simulink model "BaseEngineController_A02"  (Simulink 7.7)
settings: Solver=FixedStepDiscrete  StartTime=0.0  StopTime=10.0  RelTol=1e-3
PreLoadFcn:
  | try, eval([bdroot '_setup']); catch, eval('model_setup', ''); end
InitFcn:
  | try, eval([bdroot '_setup']); catch, eval('model_setup', ''); end
undefined symbols (not defined in the file or in its folder): ECU555, High, motohawk_build_version, motohawk_get_faults, motohawk_trunc_name, motohawk_vardec_config_group, motohawk_vardec_path, True
libraries: MotoHawk_lib x1145, AutoDoc_lib x786, motohawk_control_primitives_lib x114, motohawk_engine_lib x19, motohawk_annotations_lib x12, simulink x11, simulink_extras x1
(part 2 of 23)

== system BaseEngineController_A02/Foreground/Control/Diagnostics ==
  Control : Inport
  Sensors : Inport  Port=2
  Trigger : TriggerPort  TriggerType=function-call
  Bus Creator1 : BusCreator  Inputs=5
  Bus Selector : BusSelector  OutputSignals=Raw.RPMInst
  Constant : Constant  Value=uint8(1)
  Documentation_Calibrations_Probes_Block : AutoDoc_lib/Documentation_Calibrations_Probes_Block
  Documentation_Left_Border : AutoDoc_lib/Documentation_Left_Border  layout=Portrait
  Documentation_Right_Border : AutoDoc_lib/Documentation_Right_Border  layout=Portrait
  Documentation_Title_Block : AutoDoc_lib/Documentation_Title_Block
  Engine Overheat Fault Logic : Subsystem
  Fault Codes and States : Subsystem  TreatAsAtomicUnit=on
  Freeze Frame Trigger : Subsystem
  Freeze Frames : Subsystem
  Fuel Economy : Subsystem
  Low Oil Pressure Fault Logic : Subsystem
  Low System Voltage Fault Logic : Subsystem
  MAP Fault Logic : Subsystem
  Mux : Mux  Inputs=2
  Overspeed Protection : Subsystem  AncestorBlock=motohawk_engine_lib/Overspeed Protection
      mask Description:
      | Block allows for three levels of overspeed rev limits, and will set three fault accordingly.
      | 
      | Soft Overspeed, Medium Overspeed, and Hard Overspeed.
      mask Display:
      | patch([0 0 1 1], 0.5*[0 0.075 0.075 0], [230/255 0/255 0/255])
      | patch([0 0 1 1], 0.5*[0.025 0.05 0.05 0.025], [150/255 0/255 0/255])
      | patch([0 0 1 1], 0.5*[0 0.025 0.025 0], [0/255 0/255 0/255])
      | 
      | disp('RPM LIMITER');
  Service Intervals : Subsystem
  Terminator2 : Terminator
  Wrong Fuel Pressure Fault Logic : Subsystem
  Diagnostics : Outport
  wires:
  Service Intervals/1(HourMeter) -> Bus Creator1/2  "EngineHours"
  Bus Creator1/1 -> Diagnostics/1  "Diagnostics"
  Sensors/1 -> Bus Selector/1, Engine Overheat Fault Logic/1(Sensors), MAP Fault Logic/1(Sensors), Low Oil Pressure Fault Logic/1(Sensors), Low System Voltage Fault Logic/1(Sensors), Wrong Fuel Pressure Fault Logic/1(Sensors), Mux/2, Fuel Economy/1(Sensors)
  Control/1 -> Terminator2/1, Mux/1, Fuel Economy/2(Controller)  "Control"
  Bus Selector/1 -> Overspeed Protection/1(RPM)  "<RPMInst>"
  Freeze Frame Trigger/1(IDs) -> Freeze Frames/2(IDs)
  Freeze Frame Trigger/2(Trigger) -> Freeze Frames/3(Trigger)
  Constant/1 -> Fault Codes and States/1(IC)
  Mux/1 -> Freeze Frames/1(Data)
  Low Oil Pressure Fault Logic/1(LowOilPres) -> Bus Creator1/1  "LowOilPres"
  Engine Overheat Fault Logic/1(Overheat_Warning) -> Bus Creator1/3  "Overheat_Warning"
  Fuel Economy/1(FuelEconomy_instantaneous) -> Bus Creator1/4  "FuelEconomy_instantaneous"
  Fuel Economy/2(FuelEconomy_filtered) -> Bus Creator1/5  "FuelEconomy_filtered"

== system BaseEngineController_A02/Foreground/Control/VirtualSensors ==
  Raw : Inport
  AirCalcs : Subsystem
  Bus Creator : BusCreator  Inputs=6
  Documentation_Calibrations_Probes_Block : AutoDoc_lib/Documentation_Calibrations_Probes_Block
  Documentation_Left_Border : AutoDoc_lib/Documentation_Left_Border  layout=Portrait
  Documentation_Right_Border : AutoDoc_lib/Documentation_Right_Border  layout=Portrait
  Documentation_Title_Block : AutoDoc_lib/Documentation_Title_Block
  LoadBasedCalcs : Subsystem
  Raw Indexes : Subsystem
  RawProcessed : Subsystem
  Virtual Indexes : Subsystem
  Sensors : Outport
  wires:
  Raw/1 -> RawProcessed/1(Raw), Raw Indexes/1(Raw), AirCalcs/2(Raw), LoadBasedCalcs/2(Raw), Bus Creator/1  "Raw"
  Bus Creator/1 -> Sensors/1  "SynthSensors"
  RawProcessed/1(RawProcessed) -> Bus Creator/2, Virtual Indexes/3(RawProcessed)  "RawProcessed"
  Raw Indexes/1(RawIndexes) -> Bus Creator/3, AirCalcs/1(RawIndexes), RawProcessed/2(Indexes)  "RawIndexes"
  AirCalcs/1(AirCalcs) -> Bus Creator/4, LoadBasedCalcs/1(AirCals), Virtual Indexes/2(AirCalcs)  "AirCalcs"
  LoadBasedCalcs/1(TorqueCalcs) -> Bus Creator/5, Virtual Indexes/1(TorqueCalcs)  "TorqueCalcs"
  Virtual Indexes/1(VirtualIndexes) -> Bus Creator/6  "VirtualIndexes"

== system BaseEngineController_A02/Foreground/Inputs/CAN Inputs ==
  112 : Subsystem
  Bus Creator : BusCreator  Inputs=2
  Phoenix : Subsystem
  Out1 : Outport
  wires:
  112/1(Out1) -> Bus Creator/1  "112"
  Phoenix/1(Out1) -> Bus Creator/2  "Phoenix"
  Bus Creator/1 -> Out1/1

== system BaseEngineController_A02/Foreground/Inputs/Digital ==
  Bus Creator : BusCreator  Inputs=3
  Constant : Constant  Value=true
  Data Type Conversion : DataTypeConversion
  Documentation_Calibrations_Probes_Block : AutoDoc_lib/Documentation_Calibrations_Probes_Block
  Documentation_Left_Border : AutoDoc_lib/Documentation_Left_Border  layout=Portrait
  Documentation_Right_Border : AutoDoc_lib/Documentation_Right_Border  layout=Portrait
  Documentation_Title_Block : AutoDoc_lib/Documentation_Title_Block
  Gain : Gain  Gain=.01
  MAF_Sensor : motohawk_engine_lib/Non-Linear Sensor Characterization (tbl)  SystemSampleTime=-1  nam='MAF'  prefix='MAF'  units='g/s'  inputunits='Hz'  parent_group=motohawk_vardec_path(gcb)  tblsize=40  samp_time=-1
  Terminator1 : Terminator
  Terminator4 : Terminator
  motohawk_callout1 : motohawk_annotations_lib/motohawk_callout1  BlockType_='Mototron_harea'  desc='\fontsize{12}Read from H/W'  edit_text=Compute the mean forward body acceleration.Worry about rounding.  edit_as=255
  motohawk_callout2 : motohawk_annotations_lib/motohawk_callout1  BlockType_='Mototron_harea'  desc='\fontsize{12}Convert to Engineering Units'  edit_text=Compute the mean forward body acceleration.Worry about rounding.  edit_as=255
  motohawk_callout3 : motohawk_annotations_lib/motohawk_callout1  BlockType_='Mototron_harea'  desc='\fontsize{12}Create a Bus of Sensors'  edit_text=Compute the mean forward body acceleration.Worry about rounding.  edit_as=255
  motohawk_data_read : MotoHawk_lib/Data Storage Blocks/motohawk_data_read  source=Lookup By Name  structure=Scalar  operation=Read scalar from location by index  sz_vec=4  sz_row=2  sz_col=3  typ=boolean  nam='ESTOP'  checkNull=on  struct_inst_name='MyData'  struct_nam='MyStruct'
  motohawk_frequency_in2 : MotoHawk_lib/Advanced Digital I//O/motohawk_frequency_in  res_name=[]  text_resource=(None)  code_resource=RES_DG1M  val_resource=-1  target=ECU555-80  resource_enum_str=(None)=-1,DG1M=93,DG2M=94,DG4M=96,CNK=100  ipwm=Perform Duty Cycle Measument  itrig=off  Nmax=8  edge=Falling Edge  alias=Disabled  min_freq=10  sig=Digital Input  use_vardec=on  nam='MAF_Pin'  read_level=1  write_level=1  group='System | I/O Pins | Inputs Config'  cal_group='System | I/O Pins | Frequency In Config'
  motohawk_override_abs1 : MotoHawk_lib/Calibration & Probing Blocks/motohawk_override_abs  SystemSampleTime=-1  parent_name='ESTOP_Ovr'  parent_val=0  show_more=off  name_source=Use Parameter  parent_read_level=1  parent_override_level=1  view_as=Number  enum_struct={'Disabled', 'Enabled'}  use_help_units=off  parent_help=''  parent_units=''  use_min_max=off  parent_min=-inf  parent_max=inf  use_precision=off  parent_precision=''  parent_gain=1  parent_offset=0  parent_exp=1  use_mototune_group=off  parent_group=motohawk_vardec_path(gcb)
  motohawk_probe1 : MotoHawk_lib/Calibration & Probing Blocks/motohawk_probe  nam='ESTOP_Prb'  show_more=off  name_source=Use Parameter  read_level=1  view_as=Number  enum_struct={'Disabled', 'Enabled'}  vector_style=Wide Row  use_help_units=off  help=''  units=''  use_header_enum=off  row_header_enum={}  col_header_enum={}  use_precision=off  precision=''  gain=1  offset=0  exp=1  use_mototune_group=off  mototune_group=motohawk_vardec_path(gcb)
  SensorBus : Outport
  wires:
  Bus Creator/1 -> SensorBus/1  "Digital"
  Constant/1 -> Bus Creator/1  "GearState"
  motohawk_frequency_in2/1 -> Data Type Conversion/1
  MAF_Sensor/1 -> Bus Creator/2  "MAF"
  MAF_Sensor/2 -> Terminator4/1
  Data Type Conversion/1 -> Gain/1
  Gain/1 -> MAF_Sensor/1
  motohawk_frequency_in2/2 -> Terminator1/1
  motohawk_data_read/1 -> motohawk_override_abs1/1, motohawk_probe1/1  "EStop"
  motohawk_override_abs1/1 -> Bus Creator/3  "ESTOP"

== system BaseEngineController_A02/Foreground/Inputs/EngineSpeed ==
  note: Set PseudoRPM from MotoTune
      | to a non-zero value to cause
      | simulated RPM
  Constant : Constant  Value=0  OutDataTypeStr=Inherit: Inherit via back propagation
  Data Type Conversion : DataTypeConversion  SaturateOnIntegerOverflow=on  RndMeth=Zero
  Data Type Conversion1 : DataTypeConversion  SaturateOnIntegerOverflow=on  RndMeth=Zero
  Data Type Conversion3 : DataTypeConversion  RndMeth=Zero
  Documentation_Calibrations_Probes_Block : AutoDoc_lib/Documentation_Calibrations_Probes_Block
  Documentation_Left_Border : AutoDoc_lib/Documentation_Left_Border  layout=Portrait
  Documentation_Right_Border : AutoDoc_lib/Documentation_Right_Border  layout=Portrait
  Documentation_Title_Block : AutoDoc_lib/Documentation_Title_Block
  Encoder Diagnostics : Subsystem
  First Order Low Pass (Tunable)1 : motohawk_control_primitives_lib/First Order Low Pass (Tunable)  SystemSampleTime=-1  nam='RPMFiltConst'  samp_time=-1  group=motohawk_vardec_path(gcb)
  Gain : Gain  Gain=16  SaturateOnIntegerOverflow=on  OutDataTypeStr=Inherit: Same as input  ParamDataTypeStr=Inherit: Same as input
  Relational Operator : RelationalOperator  Operator=~=  ZeroCross=off  InputSameDT=on
  motohawk_calibration : MotoHawk_lib/Calibration & Probing Blocks/motohawk_calibration  nam='PseudoRPM'  val=0  storage=Display  show_more=on  name_source=Use Parameter  data_type=Inherit via back propagation  read_level=1  write_level=1  ovr_cals=on  view_as=Number  enum_struct={'Disabled', 'Enabled'}  vector_style=Wide Row  use_help_units=off  help=''  units=''  use_header_enum=off  row_header_enum={}  col_header_enum={}  use_min_max=off  min_val=-inf  max_val=inf  use_precision=off  precision=''  gain=1  offset=0  exp=1  use_mototune_group=on  mototune_group=motohawk_vardec_path(gcb)
  motohawk_calibration1 : MotoHawk_lib/Calibration & Probing Blocks/motohawk_calibration  nam='EngineToEncoderOffset'  val=0  storage=Calibration  show_more=on  name_source=Use Parameter  data_type=Inherit from 'Default Value'  read_level=1  write_level=1  ovr_cals=on  view_as=Number  enum_struct={'Disabled', 'Enabled'}  vector_style=Wide Row  use_help_units=on  help=''  units=''  use_header_enum=off  row_header_enum={}  col_header_enum={}  use_min_max=off  min_val=-inf  max_val=inf  use_precision=off  precision=''  gain=1  offset=0  exp=1  use_mototune_group=on  mototune_group=motohawk_vardec_path(gcb)
  motohawk_encoder_average_rpm : MotoHawk_lib/Encoder Blocks/motohawk_encoder_average_rpm
  motohawk_encoder_def : MotoHawk_lib/Encoder Blocks/motohawk_encoder_def  encoder_type=24 Minus 1 - 4-Stroke - No CAM  interface=VR Crank  sync=Rising Edge  crnkpullup=Strong Pullup  camsync=Rising Edge  campullup=Strong Pullup  angle_offset=27  sec_tdcoffset=-34.3  cam_delay=0  teeth_after_cam=-1  ignore_teeth=6  ignore_delay=100  angles=[0 270 360 630]  cnk_align_with_cam=off  adaptive_vr_cnk=off  adaptive_vr_cam=on  cal_cylinders=on  max_cylinders=8  num_cylinders=2  read_level=1  write_level=1  ovr_cals=on  group=motohawk_vardec_path(gcb)
  motohawk_encoder_fault : MotoHawk_lib/Encoder Blocks/motohawk_encoder_fault
  motohawk_encoder_instant_rpm : MotoHawk_lib/Encoder Blocks/motohawk_encoder_instant_rpm
  motohawk_encoder_offset : MotoHawk_lib/Encoder Blocks/motohawk_encoder_offset
  motohawk_encoder_pseudo : MotoHawk_lib/Encoder Blocks/motohawk_encoder_pseudo
  motohawk_encoder_state : MotoHawk_lib/Encoder Blocks/motohawk_encoder_state
  motohawk_probe : MotoHawk_lib/Calibration & Probing Blocks/motohawk_probe  nam='RPMInst'  show_more=on  name_source=Use Parameter  read_level=1  view_as=Number  enum_struct={'Disabled', 'Enabled'}  vector_style=Wide Row  use_help_units=off  help=''  units=''  use_header_enum=off  row_header_enum={}  col_header_enum={}  use_precision=off  precision=''  gain=1  offset=0  exp=1  use_mototune_group=on  mototune_group=motohawk_vardec_path(gcb)
  motohawk_probe1 : MotoHawk_lib/Calibration & Probing Blocks/motohawk_probe  nam='RPM'  show_more=on  name_source=Use Parameter  read_level=1  view_as=Number  enum_struct={'Disabled', 'Enabled'}  vector_style=Wide Row  use_help_units=on  help=''  units=''  use_header_enum=off  row_header_enum={}  col_header_enum={}  use_precision=on  precision=''  gain=1  offset=0  exp=1  use_mototune_group=on  mototune_group=motohawk_vardec_path(gcb)
  motohawk_probe2 : MotoHawk_lib/Calibration & Probing Blocks/motohawk_probe  nam='EncoderState'  show_more=on  name_source=Use Parameter  read_level=1  view_as=Enumeration  enum_struct={'zero','ZERO_SPEED', 'POSN_UNKNOWN','ENC_SYNC_CAM_UNKNOWN','ENC_SYNC_CAM_SYNC'}  vector_style=Wide Row  use_help_units=off  help=''  units=''  use_header_enum=off  row_header_enum={}  col_header_enum={}  use_precision=off  precision=''  gain=1  offset=0  exp=1  use_mototune_group=on  mototune_group=motohawk_vardec_path(gcb)
  RPMInst : Outport
  RPM : Outport  Port=2
  wires:
  First Order Low Pass (Tunable)1/1 -> motohawk_probe1/1, RPM/1  "RPM"
  motohawk_encoder_fault/1 -> Encoder Diagnostics/1(Encoder Fault)
  motohawk_encoder_state/1 -> motohawk_probe2/1
  Data Type Conversion3/1 -> motohawk_encoder_offset/1
  Gain/1 -> Data Type Conversion3/1
  motohawk_calibration1/1 -> Gain/1
  Data Type Conversion1/1 -> motohawk_probe/1, RPMInst/1  "RPMInst"
  Data Type Conversion/1 -> First Order Low Pass (Tunable)1/1  "RPM"
  Constant/1 -> Relational Operator/1
  Relational Operator/1 -> motohawk_encoder_pseudo/1
  motohawk_calibration/1 -> Relational Operator/2, motohawk_encoder_pseudo/2
  motohawk_encoder_average_rpm/1 -> Data Type Conversion/1  "RPM"
  motohawk_encoder_instant_rpm/1 -> Data Type Conversion1/1  "RPMInst"

== system BaseEngineController_A02/Foreground/Inputs/LinearSensors ==
  Bus Creator3 : BusCreator  Inputs=9
  Documentation_Calibrations_Probes_Block : AutoDoc_lib/Documentation_Calibrations_Probes_Block
  Documentation_Left_Border : AutoDoc_lib/Documentation_Left_Border  layout=Portrait
  Documentation_Right_Border : AutoDoc_lib/Documentation_Right_Border  layout=Portrait
  Documentation_Title_Block : AutoDoc_lib/Documentation_Title_Block
  EGRSensor1 : motohawk_engine_lib/Linear Sensor Characterization  SystemSampleTime=-1  nam='EGRSense'  prefix='EGRSense'  units='%'  inputunits='V'  parent_group=motohawk_vardec_path(gcb)  samp_time=-1
  FuelOxygenationSensor : motohawk_engine_lib/Linear Sensor Characterization  SystemSampleTime=-1  nam='FuelSens'  prefix='FuelSens'  units='%'  inputunits='Hz'  parent_group=motohawk_vardec_path(gcb)  samp_time=-1
  FuelPres : motohawk_engine_lib/Linear Sensor Characterization  SystemSampleTime=-1  nam='FuelPres'  prefix='FuelPres'  units='kPa Gauge'  inputunits='ADC'  parent_group=motohawk_vardec_path(gcb)  samp_time=-1
  Gain : Gain  Gain=100  OutDataTypeStr=double
  MAP : motohawk_engine_lib/Linear Sensor Characterization  SystemSampleTime=-1  nam='MAP'  prefix='MAP'  units='kPa'  inputunits='ADC'  parent_group=motohawk_vardec_path(gcb)  samp_time=-1
  MAPTime : motohawk_engine_lib/Linear Sensor Characterization  SystemSampleTime=-1  nam='MAPTime'  prefix='MAPTime'  units='kPa'  inputunits='ADC'  parent_group=motohawk_vardec_path(gcb)  samp_time=-1
  MAP_Crank_Synchronous : Subsystem
      mask Display: disp('Crank-Synchronous MAP');
  OilPres : motohawk_engine_lib/Linear Sensor Characterization  SystemSampleTime=-1  nam='OilPres'  prefix='OilPres'  units='psi'  inputunits='ADC'  parent_group=motohawk_vardec_path(gcb)  samp_time=-1
  SensVolts : motohawk_engine_lib/Linear Sensor Characterization  SystemSampleTime=-1  nam='SensVolt'  prefix='SensVolt'  units='Volts'  inputunits='ADC'  parent_group=motohawk_vardec_path(gcb)  samp_time=-1
  SysVolts : motohawk_engine_lib/Linear Sensor Characterization  SystemSampleTime=-1  nam='SysVolt'  prefix='SysVolt'  units='Volts'  inputunits='ADC'  parent_group=motohawk_vardec_path(gcb)  samp_time=-1
  Terminator{1..7} (7 alike) : Terminator
  motohawk_ain1 : MotoHawk_lib/Analog I//O Blocks/motohawk_ain  res_name=[]  text_resource=(None)  code_resource=RES_ECUP  val_resource=-1  target=ECU555-80  resource_enum_str=(None)=-1,ECUP=53,DRVP=54,XDRP=55,AN1M=56,AN2M=57,AN3M=58,AN4M=59,AN5M=60,AN6M=61,AN7M=62,AN8M=63,AN9M=64,AN10M=65,AN11M=66,AN12M=67,AN13M=68,AN14M=69,AN15M=70,AN16M=71,AN17M=72,AN18M=73,AN19M=74,O2A=90,O2B=91,XDRP_B=92  altref=5V Reference  typ=uint16  num_adc_bits=10  use_stat=off  use_vardec=on  nam='OilPresPin'  read_level=1  write_level=1  group='System | I/O Pins | Inputs Config'
  motohawk_ain14 : MotoHawk_lib/Analog I//O Blocks/motohawk_ain  res_name=[]  text_resource=DRVP  code_resource=RES_ECUP  val_resource=-1  target=ECU555-80  resource_enum_str=(None)=-1,ECUP=53,DRVP=54,XDRP=55,AN1M=56,AN2M=57,AN3M=58,AN4M=59,AN5M=60,AN6M=61,AN7M=62,AN8M=63,AN9M=64,AN10M=65,AN11M=66,AN12M=67,AN13M=68,AN14M=69,AN15M=70,AN16M=71,AN17M=72,AN18M=73,AN19M=74,O2A=90,O2B=91,XDRP_B=92  altref=5V Reference  typ=uint16  num_adc_bits=10  use_stat=off  use_vardec=on  nam='SysVoltage'  read_level=1  write_level=1  group='System | I/O Pins | Inputs Config'
  motohawk_ain2 : MotoHawk_lib/Analog I//O Blocks/motohawk_ain  res_name=[]  text_resource=(None)  code_resource=RES_ECUP  val_resource=-1  target=ECU555-80  resource_enum_str=(None)=-1,ECUP=53,DRVP=54,XDRP=55,AN1M=56,AN2M=57,AN3M=58,AN4M=59,AN5M=60,AN6M=61,AN7M=62,AN8M=63,AN9M=64,AN10M=65,AN11M=66,AN12M=67,AN13M=68,AN14M=69,AN15M=70,AN16M=71,AN17M=72,AN18M=73,AN19M=74,O2A=90,O2B=91,XDRP_B=92  altref=5V Reference  typ=uint16  num_adc_bits=10  use_stat=off  use_vardec=on  nam='FuelPresPin'  read_level=1  write_level=1  group='System | I/O Pins | Inputs Config'
  motohawk_ain3 : MotoHawk_lib/Analog I//O Blocks/motohawk_ain  res_name=[]  text_resource=XDRP  code_resource=RES_ECUP  val_resource=-1  target=ECU555-80  resource_enum_str=(None)=-1,ECUP=53,DRVP=54,XDRP=55,AN1M=56,AN2M=57,AN3M=58,AN4M=59,AN5M=60,AN6M=61,AN7M=62,AN8M=63,AN9M=64,AN10M=65,AN11M=66,AN12M=67,AN13M=68,AN14M=69,AN15M=70,AN16M=71,AN17M=72,AN18M=73,AN19M=74,O2A=90,O2B=91,XDRP_B=92  altref=5V Reference  typ=uint16  num_adc_bits=10  use_stat=off  use_vardec=on  nam='SensVoltSensorPin'  read_level=1  write_level=1  group='System | I/O Pins | Inputs Config'
  motohawk_ain4 : MotoHawk_lib/Analog I//O Blocks/motohawk_ain  res_name=[]  text_resource=(None)  code_resource=RES_ECUP  val_resource=-1  target=ECU555-80  resource_enum_str=(None)=-1,ECUP=53,DRVP=54,XDRP=55,AN1M=56,AN2M=57,AN3M=58,AN4M=59,AN5M=60,AN6M=61,AN7M=62,AN8M=63,AN9M=64,AN10M=65,AN11M=66,AN12M=67,AN13M=68,AN14M=69,AN15M=70,AN16M=71,AN17M=72,AN18M=73,AN19M=74,O2A=90,O2B=91,XDRP_B=92  altref=5V Reference  typ=uint16  num_adc_bits=10  use_stat=off  use_vardec=on  nam='EGRPin'  read_level=1  write_level=1  group='System | I/O Pins | Inputs Config'
  motohawk_ain_read1 : MotoHawk_lib/Analog I//O Blocks/motohawk_ain_read  nam='MAPSensorPin'  typ=uint16
  motohawk_callout1 : motohawk_annotations_lib/motohawk_callout1  BlockType_='Mototron_harea'  desc='\fontsize{12}Read from H/W'  edit_text=Compute the mean forward body acceleration.Worry about rounding.  edit_as=255
  motohawk_callout2 : motohawk_annotations_lib/motohawk_callout1  BlockType_='Mototron_harea'  desc='\fontsize{12}Convert to Engineering Units'  edit_text=Compute the mean forward body acceleration.Worry about rounding.  edit_as=255
  motohawk_callout3 : motohawk_annotations_lib/motohawk_callout1  BlockType_='Mototron_harea'  desc='\fontsize{12}Create a Bus of Sensors'  edit_text=Compute the mean forward body acceleration.Worry about rounding.  edit_as=255
  motohawk_frequency_in : MotoHawk_lib/Advanced Digital I//O/motohawk_frequency_in  res_name=[]  text_resource=DG1M  code_resource=RES_DG1M  val_resource=-1  target=ECU555-80  resource_enum_str=(None)=-1,DG1M=93,DG2M=94,DG4M=96,CNK=100  ipwm=No Duty Cycle Measurement  itrig=off  Nmax=10  edge=Rising Edge  alias=Disabled  min_freq=10  sig=Digital Input  use_vardec=on  nam='FuelSensorPin'  read_level=1  write_level=1  group='System | I/O Pins | Inputs Config'  cal_group='System | I/O Pins | Frequency In Config'
  SensorBus : Outport
  wires:
  Bus Creator3/1 -> SensorBus/1  "LinearSensors"
  motohawk_ain_read1/1 -> MAPTime/1
  MAP_Crank_Synchronous/1(ADC) -> MAP/1
  SysVolts/2 -> Terminator4/1
  SensVolts/2 -> Terminator3/1
  MAP/2 -> Terminator2/1
  MAPTime/2 -> Bus Creator3/5  "MAP_Time_Raw"
  MAPTime/1 -> Bus Creator3/4  "Map_Time"
  MAP/1 -> Bus Creator3/3  "MAP_Angular"
  SensVolts/1 -> Bus Creator3/2  "SensVolt"
  motohawk_ain3/1 -> SensVolts/1
  SysVolts/1 -> Bus Creator3/1  "SysVolt"
  motohawk_ain14/1 -> SysVolts/1
  motohawk_ain1/1 -> OilPres/1
  OilPres/2 -> Terminator1/1
  OilPres/1 -> Bus Creator3/6  "OilPres"
  motohawk_frequency_in/1 -> Gain/1
  FuelOxygenationSensor/2 -> Terminator6/1
  motohawk_ain2/1 -> FuelPres/1
  FuelPres/2 -> Terminator7/1
  FuelOxygenationSensor/1 -> Bus Creator3/7  "FuelSense"
  FuelPres/1 -> Bus Creator3/8  "FuelPres"
  Gain/1 -> FuelOxygenationSensor/1
  EGRSensor1/2 -> Terminator5/1
  motohawk_ain4/1 -> EGRSensor1/1
  EGRSensor1/1 -> Bus Creator3/9  "EGRPos"

== system BaseEngineController_A02/Foreground/Inputs/NonLinearSensors ==
  Bus Creator1 : BusCreator  Inputs=6
  Documentation_Calibrations_Probes_Block : AutoDoc_lib/Documentation_Calibrations_Probes_Block
  Documentation_Left_Border : AutoDoc_lib/Documentation_Left_Border  layout=Portrait
  Documentation_Right_Border : AutoDoc_lib/Documentation_Right_Border  layout=Portrait
  Documentation_Title_Block : AutoDoc_lib/Documentation_Title_Block
  O2_PostCat : Subsystem  AncestorBlock=motohawk_engine_lib/Non-Linear Sensor Characterization (tbl)  mask(Non-Linear Sensor Characterization (tbl)){nam='O2_PostCat', prefix='O2_PostCat', units='Equiv Ratio', inputunits='ADC', parent_group=motohawk_vardec_path(gcb), bp=[12 17 25 30 37 42 48 71 100 130 168 202 256 281 300 323 338 361 379 380], data=[0.75 0.86 0.93 0.96 0.98 0.99 0.995 0.998 0.999 1 1.001 1.002 1.005 1.01 1.02 1.04 1.06 1.1 1.15 1.25], min=1, max=425, default=1, filt_const=.005, samp_time=-1}
      mask prompts: nam "Name"; units "Output Units"; inputunits "Input Units"; parent_group "MotoTune Group"; bp "Breakpoints"; data "Table Data"; min "Min Fault Thresh"; max "Max Fault Thresh"; default "Initial Default Value When Fault Suspected"; filt_const "1st-Order Filter Constant"; samp_time "Sample Time"
      mask help:
      | Converts from A/D Counts to Engineering Units using a 1-D Table Lookup.
      | Saturates the converted value around Min and Max values and triggers a Fault when out of range.
      mask init:
      | lbl = ['Non-Linear Sensor (tbl): ' nam ' [' units ']'];
      | group = [parent_group ' | ' nam];
      settings: RTWSystemCode=Function  RTWFcnNameOpts=Use subsystem name  RTWFileNameOpts=Use function name
      mask Display:
      | patch([0 0 1 1], [0 0.075 0.075 0], [230/255 0/255 0/255])
      | patch([0 0 1 1], [0.025 0.05 0.05 0.025], [150/255 0/255 0/255])
      | patch([0 0 1 1], [0 0.025 0.025 0], [0/255 0/255 0/255])
      | 
      | disp(lbl);
  Temp Sensors : Subsystem  TreatAsAtomicUnit=on
  Terminator2 : Terminator
  motohawk_ain3 : MotoHawk_lib/Analog I//O Blocks/motohawk_ain  res_name=[]  text_resource=AN12M  code_resource=RES_ECUP  val_resource=-1  target=ECU555-80  resource_enum_str=(None)=-1,ECUP=53,DRVP=54,XDRP=55,AN1M=56,AN2M=57,AN3M=58,AN4M=59,AN5M=60,AN6M=61,AN7M=62,AN8M=63,AN9M=64,AN10M=65,AN11M=66,AN12M=67,AN13M=68,AN14M=69,AN15M=70,AN16M=71,AN17M=72,AN18M=73,AN19M=74,O2A=90,O2B=91,XDRP_B=92  altref=5V Reference  typ=uint16  num_adc_bits=10  use_stat=off  use_vardec=on  nam='PostO2Pin'  read_level=1  write_level=1  group='System | I/O Pins | Inputs Config'
  motohawk_callout1 : motohawk_annotations_lib/motohawk_callout1  BlockType_='Mototron_harea'  desc='\fontsize{12}Read from H/W'  edit_text=Compute the mean forward body acceleration.Worry about rounding.  edit_as=255
  motohawk_callout2 : motohawk_annotations_lib/motohawk_callout1  BlockType_='Mototron_harea'  desc='\fontsize{12}Convert to Engineering Units'  edit_text=Compute the mean forward body acceleration.Worry about rounding.  edit_as=255
  motohawk_callout3 : motohawk_annotations_lib/motohawk_callout1  BlockType_='Mototron_harea'  desc='\fontsize{12}Create a Bus of Sensors'  edit_text=Compute the mean forward body acceleration.Worry about rounding.  edit_as=255
  motohawk_trigger : MotoHawk_lib/Trigger Blocks/motohawk_trigger  Tag=Trigger  trigger=FGND_5XRTI_PERIODIC  function_name=Trigger_FGND_5XRTI_PERIODIC_8397p0001  priority_order=1  inherited=on  run_disabled=off  override_app_monitor=off  target=ECU555_80
  SensorBus : Outport
  wires:
  Bus Creator1/1 -> SensorBus/1  "NonLinearSensors"
  Temp Sensors/2(ECT_Raw) -> Bus Creator1/2  "ECT_Raw"
  Temp Sensors/4(IAT_Raw) -> Bus Creator1/4  "IAT_Raw"
  Temp Sensors/3(IAT) -> Bus Creator1/3  "IAT"
  Temp Sensors/1(ECT) -> Bus Creator1/1  "ECT"
  motohawk_ain3/1 -> O2_PostCat/1(ADC)
  O2_PostCat/2(EngUnitsRaw) -> Terminator2/1
  motohawk_trigger/1 -> Temp Sensors/Trigger
  O2_PostCat/1(EngUnitsFilt) -> Bus Creator1/5  "O2_PostCat"
  O2_PostCat/3(O2_ADC) -> Bus Creator1/6  "O2_PostCat_ADC"

== system BaseEngineController_A02/Foreground/Inputs/PotentiometerSensors ==
  Bus Creator1 : BusCreator
  Constant4 : Constant  Value=false
  Constant5 : Constant  Value=false
  Documentation_Calibrations_Probes_Block : AutoDoc_lib/Documentation_Calibrations_Probes_Block
  Documentation_Left_Border : AutoDoc_lib/Documentation_Left_Border  layout=Portrait
  Documentation_Right_Border : AutoDoc_lib/Documentation_Right_Border  layout=Portrait
  Documentation_Title_Block : AutoDoc_lib/Documentation_Title_Block
  TPS1 : motohawk_engine_lib/Linear Sensor Characterization w Adapt  SystemSampleTime=-1  nam='TPS1'  prefix='TPS1'  samp_time=-1  parent_group=motohawk_vardec_path(gcb)
  TPS2 : motohawk_engine_lib/Linear Sensor Characterization w Adapt  SystemSampleTime=-1  nam='TPS2'  prefix='TPS2'  samp_time=-1  parent_group=motohawk_vardec_path(gcb)
  Terminator10 : Terminator
  Terminator11 : Terminator
  motohawk_ain6 : MotoHawk_lib/Analog I//O Blocks/motohawk_ain  res_name=[]  text_resource=AN2M  code_resource=RES_ECUP  val_resource=-1  target=ECU555-80  resource_enum_str=(None)=-1,ECUP=53,DRVP=54,XDRP=55,AN1M=56,AN2M=57,AN3M=58,AN4M=59,AN5M=60,AN6M=61,AN7M=62,AN8M=63,AN9M=64,AN10M=65,AN11M=66,AN12M=67,AN13M=68,AN14M=69,AN15M=70,AN16M=71,AN17M=72,AN18M=73,AN19M=74,O2A=90,O2B=91,XDRP_B=92  altref=5V Reference  typ=uint16  num_adc_bits=10  use_stat=off  use_vardec=on  nam='TPS1AnalogInput'  read_level=1  write_level=1  group=motohawk_vardec_path(gcb)
  motohawk_ain9 : MotoHawk_lib/Analog I//O Blocks/motohawk_ain  res_name=[]  text_resource=AN3M  code_resource=RES_ECUP  val_resource=-1  target=ECU555-80  resource_enum_str=(None)=-1,ECUP=53,DRVP=54,XDRP=55,AN1M=56,AN2M=57,AN3M=58,AN4M=59,AN5M=60,AN6M=61,AN7M=62,AN8M=63,AN9M=64,AN10M=65,AN11M=66,AN12M=67,AN13M=68,AN14M=69,AN15M=70,AN16M=71,AN17M=72,AN18M=73,AN19M=74,O2A=90,O2B=91,XDRP_B=92  altref=5V Reference  typ=uint16  num_adc_bits=10  use_stat=off  use_vardec=on  nam='TPS2AnalogInput'  read_level=1  write_level=1  group=motohawk_vardec_path(gcb)
  motohawk_callout1 : motohawk_annotations_lib/motohawk_callout1  BlockType_='Mototron_harea'  desc='\fontsize{12}Read from H/W'  edit_text=Compute the mean forward body acceleration.Worry about rounding.  edit_as=255
  motohawk_callout2 : motohawk_annotations_lib/motohawk_callout1  BlockType_='Mototron_harea'  desc='\fontsize{12}Convert to Engineering Units'  edit_text=Compute the mean forward body acceleration.Worry about rounding.  edit_as=255
  motohawk_callout3 : motohawk_annotations_lib/motohawk_callout1  BlockType_='Mototron_harea'  desc='\fontsize{12}Create a Bus of Sensors'  edit_text=Compute the mean forward body acceleration.Worry about rounding.  edit_as=255
  motohawk_data_read : MotoHawk_lib/Data Storage Blocks/motohawk_data_read  source=Lookup By Name  structure=Scalar  operation=Read scalar from location by index  sz_vec=4  sz_row=2  sz_col=3  typ=boolean  nam='TPSAdaptLoRequest'  checkNull=on  struct_inst_name='Struct1'  struct_nam='MyStruct'
  motohawk_data_read1 : MotoHawk_lib/Data Storage Blocks/motohawk_data_read  source=Lookup By Name  structure=Scalar  operation=Read scalar from location by index  sz_vec=4  sz_row=2  sz_col=3  typ=double  nam='APP'  checkNull=on  struct_inst_name='MyData'  struct_nam='MyStruct'
  motohawk_override_abs1 : MotoHawk_lib/Calibration & Probing Blocks/motohawk_override_abs  SystemSampleTime=-1  parent_name='APP2_Ovr'  parent_val=0  show_more=off  name_source=Use Parameter  parent_read_level=1  parent_override_level=1  view_as=Number  enum_struct={'Disabled', 'Enabled'}  use_help_units=off  parent_help=''  parent_units=''  use_min_max=off  parent_min=-inf  parent_max=inf  use_precision=off  parent_precision=''  parent_gain=1  parent_offset=0  parent_exp=1  use_mototune_group=off  parent_group=motohawk_vardec_path(gcb)
  motohawk_override_abs13 : MotoHawk_lib/Calibration & Probing Blocks/motohawk_override_abs  SystemSampleTime=-1  parent_name='APP1_Ovr'  parent_val=0  show_more=off  name_source=Use Parameter  parent_read_level=1  parent_override_level=1  view_as=Number  enum_struct={'Disabled', 'Enabled'}  use_help_units=off  parent_help=''  parent_units=''  use_min_max=off  parent_min=-inf  parent_max=inf  use_precision=off  parent_precision=''  parent_gain=1  parent_offset=0  parent_exp=1  use_mototune_group=off  parent_group=motohawk_vardec_path(gcb)
  motohawk_probe1 : MotoHawk_lib/Calibration & Probing Blocks/motohawk_probe  nam='APP2_Prb'  show_more=off  name_source=Use Parameter  read_level=1  view_as=Number  enum_struct={'Disabled', 'Enabled'}  vector_style=Wide Row  use_help_units=off  help=''  units=''  use_header_enum=off  row_header_enum={}  col_header_enum={}  use_precision=off  precision=''  gain=1  offset=0  exp=1  use_mototune_group=off  mototune_group=motohawk_vardec_path(gcb)
  motohawk_probe13 : MotoHawk_lib/Calibration & Probing Blocks/motohawk_probe  nam='APP1_Prb'  show_more=off  name_source=Use Parameter  read_level=1  view_as=Number  enum_struct={'Disabled', 'Enabled'}  vector_style=Wide Row  use_help_units=off  help=''  units=''  use_header_enum=off  row_header_enum={}  col_header_enum={}  use_precision=off  precision=''  gain=1  offset=0  exp=1  use_mototune_group=off  mototune_group=motohawk_vardec_path(gcb)
  SensorBus : Outport
  wires:
  Constant5/1 -> TPS2/3
  Constant4/1 -> TPS1/3
  motohawk_data_read/1 -> TPS2/2, TPS1/2
  Bus Creator1/1 -> SensorBus/1  "PotSensors"
  TPS2/2 -> Terminator11/1
  TPS1/2 -> Terminator10/1
  motohawk_ain9/1 -> TPS2/1
  motohawk_ain6/1 -> TPS1/1
  TPS1/1 -> Bus Creator1/3  "TPS1"
  TPS2/1 -> Bus Creator1/4  "TPS2"
  motohawk_override_abs13/1 -> Bus Creator1/1  "APP1"
  motohawk_data_read1/1 -> motohawk_override_abs13/1, motohawk_probe13/1, motohawk_override_abs1/1, motohawk_probe1/1
  motohawk_override_abs1/1 -> Bus Creator1/2  "APP2"

== system BaseEngineController_A02/Foreground/Outputs/CAN Out ==
  In1 : Inport
  112 : Subsystem
  Phoenix : Subsystem
  wires:
  In1/1 -> Phoenix/1(In1), 112/1(In1)

== system BaseEngineController_A02/Foreground/Outputs/Coil Control ==
  SparkEnable : Inport
  SparkStartAngle : Inport  Port=2
  SparkAdvance : Inport  Port=3
  CutSequence : Inport  Port=4
  Trigger : TriggerPort  TriggerType=function-call
  Coil Enable Manager : Subsystem
  Data Type Conversion : DataTypeConversion  SaturateOnIntegerOverflow=on  RndMeth=Zero
  Data Type Conversion1 : DataTypeConversion  SaturateOnIntegerOverflow=on  RndMeth=Zero
  Data Type Conversion2 : DataTypeConversion  SaturateOnIntegerOverflow=on  RndMeth=Zero
  Documentation_Calibrations_Probes_Block : AutoDoc_lib/Documentation_Calibrations_Probes_Block
  Documentation_Left_Border : AutoDoc_lib/Documentation_Left_Border  layout=Portrait
  Documentation_Right_Border : AutoDoc_lib/Documentation_Right_Border  layout=Portrait
  Documentation_Title_Block : AutoDoc_lib/Documentation_Title_Block
  EST Fault Management : Subsystem
  Gain : Gain  Gain=16  SaturateOnIntegerOverflow=on  OutDataTypeStr=Inherit: Same as input  ParamDataTypeStr=Inherit: Same as input
  Gain1 : Gain  Gain=16  SaturateOnIntegerOverflow=on  OutDataTypeStr=Inherit: Same as input  ParamDataTypeStr=Inherit: Same as input
  Gain2 : Gain  Gain=1000  SaturateOnIntegerOverflow=on  OutDataTypeStr=Inherit: Same as input  ParamDataTypeStr=Inherit: Same as input
  Logical Operator1 : Logic  Inputs=3  AllPortsSameDT=off  OutDataTypeStr=boolean
  Spark Sequence : MotoHawk_lib/Advanced Digital I//O/Spark Sequence  text_resource=EST 1  code_resource=RES_EST1  val_resource=-1  target=ECU555-80  resource_enum_str=(None)=-1,EST 1=36,EST 2=37,EST 3=38,EST 4=39,EST 5=40,EST 6=41,EST 7=42,EST 8=43  sequence_type=Batch (Wasted spark)  stop_mode=Angle Controlled  update_mode=OS  granularity=Fine  min_duration_mode=Static  min_duration=1.5  use_diag=on  open_threshold=500  use_vardec=on  nam='EST_'  read_level=1  write_level=1  group='System | I/O Pins | Outputs Config'
  motohawk_calibration2 : MotoHawk_lib/Calibration & Probing Blocks/motohawk_calibration  nam='SparkMaxDuration'  val=5  storage=Calibration  show_more=on  name_source=Use Parameter  data_type=Inherit via back propagation  read_level=1  write_level=1  ovr_cals=on  view_as=Number  enum_struct={'Disabled', 'Enabled'}  vector_style=Wide Row  use_help_units=on  help="'Maximum duration for a spark pulse.  Must be a multiple of 5ms'"  units='ms'  use_header_enum=off  row_header_enum={}  col_header_enum={}  use_min_max=off  min_val=-inf  max_val=inf  use_precision=off  precision=''  gain=1  offset=0  exp=1  use_mototune_group=on  mototune_group=motohawk_vardec_path(gcb)
  motohawk_override_rel : MotoHawk_lib/Calibration & Probing Blocks/motohawk_override_rel  SystemSampleTime=-1  parent_name='SparkStartAngle'  show_more=on  name_source=Use Parameter  parent_read_level=1  parent_override_level=1  use_help_units=on  parent_help='Provides a relative offset to input signal'  parent_units='degBTDC'  use_min_max=off  parent_min=-inf  parent_max=inf  use_precision=off  parent_precision=''  parent_gain=1  parent_offset=0  parent_exp=1  use_mototune_group=on  parent_group=motohawk_vardec_path(gcb)
  motohawk_override_rel1 : MotoHawk_lib/Calibration & Probing Blocks/motohawk_override_rel  SystemSampleTime=-1  parent_name='SparkStopAngle'  show_more=on  name_source=Use Parameter  parent_read_level=1  parent_override_level=1  use_help_units=on  parent_help='Provides a relative offset to input signal'  parent_units='degBTDC'  use_min_max=off  parent_min=-inf  parent_max=inf  use_precision=off  parent_precision=''  parent_gain=1  parent_offset=0  parent_exp=1  use_mototune_group=on  parent_group=motohawk_vardec_path(gcb)
  motohawk_probe : MotoHawk_lib/Calibration & Probing Blocks/motohawk_probe  nam='CoilStartAngle'  show_more=on  name_source=Use Parameter  read_level=1  view_as=Number  enum_struct={'Disabled', 'Enabled'}  vector_style=Wide Row  use_help_units=on  help='StartAngle for coils to establish dwell.'  units='degBTDC'  use_header_enum=off  row_header_enum={}  col_header_enum={}  use_precision=off  precision=''  gain=1  offset=0  exp=1  use_mototune_group=on  mototune_group=motohawk_vardec_path(gcb)
  motohawk_probe1 : MotoHawk_lib/Calibration & Probing Blocks/motohawk_probe  nam='CoilStopAngle'  show_more=on  name_source=Use Parameter  read_level=1  view_as=Number  enum_struct={'Disabled', 'Enabled'}  vector_style=Wide Row  use_help_units=on  help='Stop angle for coils to establish dwell.'  units='degBTDC'  use_header_enum=off  row_header_enum={}  col_header_enum={}  use_precision=off  precision=''  gain=1  offset=0  exp=1  use_mototune_group=on  mototune_group=motohawk_vardec_path(gcb)
  motohawk_probe2 : MotoHawk_lib/Calibration & Probing Blocks/motohawk_probe  nam='CoilEnabled'  show_more=on  name_source=Use Parameter  read_level=1  view_as=Number  enum_struct={'Disabled', 'Enabled'}  vector_style=Wide Row  use_help_units=on  help='Enable sate for coils.'  units='bool'  use_header_enum=off  row_header_enum={}  col_header_enum={}  use_precision=off  precision=''  gain=1  offset=0  exp=1  use_mototune_group=on  mototune_group=motohawk_vardec_path(gcb)
  wires:
  CutSequence/1 -> Logical Operator1/3
  SparkEnable/1 -> Logical Operator1/2
  Coil Enable Manager/1(Enable) -> Logical Operator1/1
  Gain2/1 -> Data Type Conversion2/1
  Data Type Conversion2/1 -> Spark Sequence/4
  Spark Sequence/4 -> EST Fault Management/4(Dwell)
  Spark Sequence/3 -> EST Fault Management/3(OpenADC)
  Spark Sequence/2 -> EST Fault Management/2(Open)
  Spark Sequence/1 -> EST Fault Management/1(Short)
  motohawk_override_rel/1 -> motohawk_probe/1, Gain/1
  motohawk_override_rel1/1 -> motohawk_probe1/1, Gain1/1
  Logical Operator1/1 -> motohawk_probe2/1, Spark Sequence/1
  SparkAdvance/1 -> motohawk_override_rel1/1
  SparkStartAngle/1 -> motohawk_override_rel/1
  motohawk_calibration2/1 -> Gain2/1
  Data Type Conversion1/1 -> Spark Sequence/3
  Data Type Conversion/1 -> Spark Sequence/2
  Gain1/1 -> Data Type Conversion1/1
  Gain/1 -> Data Type Conversion/1

== system BaseEngineController_A02/Foreground/Outputs/EGR Out ==
  EGR : Inport
  Bus Selector : BusSelector  OutputSignals=ActuatorCharacterization.EGR.Enable,ActuatorCharacterization.EGR.Duration
  Constant : Constant  Value=0  OutDataTypeStr=boolean
  Data Type Conversion : DataTypeConversion  OutDataTypeStr=int32
  motohawk_oneshot : MotoHawk_lib/Advanced Digital I//O/motohawk_oneshot  res_nam=[]  text_resource=ESC  code_resource=NONE  val_resource=-1  target=ECU555-80  resource_enum_str=(None)=-1,HSOL1=2,HSOL2=3,HSOL3=4,HSOL4=5,START=6,OILP=11,ESC=35,EST 1=36,EST 2=37,EST 3=38,EST 4=39,EST 5=40,EST 6=41,EST 7=42,EST 8=43  show_ports=on  use_vardec=on  nam='ONESHOT'  read_level=1  write_level=1  group='System | I/O Pins | Outputs Config'
  motohawk_override_abs : MotoHawk_lib/Calibration & Probing Blocks/motohawk_override_abs  SystemSampleTime=-1  parent_name='Trigger_Ovr'  parent_val=0  show_more=off  name_source=Use Parameter  parent_read_level=1  parent_override_level=1  view_as=Number  enum_struct={'Disabled', 'Enabled'}  use_help_units=off  parent_help=''  parent_units=''  use_min_max=off  parent_min=-inf  parent_max=inf  use_precision=off  parent_precision=''  parent_gain=1  parent_offset=0  parent_exp=1  use_mototune_group=off  parent_group=motohawk_vardec_path(gcb)
  motohawk_override_abs1 : MotoHawk_lib/Calibration & Probing Blocks/motohawk_override_abs  SystemSampleTime=-1  parent_name='Duration_Ovr'  parent_val=0  show_more=off  name_source=Use Parameter  parent_read_level=1  parent_override_level=1  view_as=Number  enum_struct={'Disabled', 'Enabled'}  use_help_units=off  parent_help=''  parent_units=''  use_min_max=off  parent_min=-inf  parent_max=inf  use_precision=off  parent_precision=''  parent_gain=1  parent_offset=0  parent_exp=1  use_mototune_group=off  parent_group=motohawk_vardec_path(gcb)
  motohawk_probe : MotoHawk_lib/Calibration & Probing Blocks/motohawk_probe  nam='Trigger_Prb'  show_more=off  name_source=Use Parameter  read_level=1  view_as=Number  enum_struct={'Disabled', 'Enabled'}  vector_style=Wide Row  use_help_units=off  help=''  units=''  use_header_enum=off  row_header_enum={}  col_header_enum={}  use_precision=off  precision=''  gain=1  offset=0  exp=1  use_mototune_group=off  mototune_group=motohawk_vardec_path(gcb)
  motohawk_probe1 : MotoHawk_lib/Calibration & Probing Blocks/motohawk_probe  nam='Duration_Prb'  show_more=off  name_source=Use Parameter  read_level=1  view_as=Number  enum_struct={'Disabled', 'Enabled'}  vector_style=Wide Row  use_help_units=off  help=''  units=''  use_header_enum=off  row_header_enum={}  col_header_enum={}  use_precision=off  precision=''  gain=1  offset=0  exp=1  use_mototune_group=off  mototune_group=motohawk_vardec_path(gcb)
  wires:
  EGR/1 -> Bus Selector/1
  motohawk_override_abs/1 -> motohawk_oneshot/1
  Bus Selector/1 -> motohawk_override_abs/1, motohawk_probe/1  "<Enable>"
  motohawk_override_abs1/1 -> motohawk_oneshot/2
  Bus Selector/2 -> Data Type Conversion/1  "<Duration>"
  Constant/1 -> motohawk_oneshot/3
  Data Type Conversion/1 -> motohawk_override_abs1/1, motohawk_probe1/1

== system BaseEngineController_A02/Foreground/Outputs/FuelPump ==
  State : Inport
  CompareTo : motohawk_control_primitives_lib/CompareTo  SystemSampleTime=-1  op===  val=1
  Discrete Actuator - FUELP : motohawk_engine_lib/Discrete Actuator Characterization  SystemSampleTime=-1  prefix='FUELP'  nam='FUELP'  units='bool'  parent_group=motohawk_vardec_path(gcb)  polarity=High-True
  Documentation_Calibrations_Probes_Block : AutoDoc_lib/Documentation_Calibrations_Probes_Block
  Documentation_Left_Border : AutoDoc_lib/Documentation_Left_Border  layout=Portrait
  Documentation_Right_Border : AutoDoc_lib/Documentation_Right_Border  layout=Portrait
  Documentation_Title_Block : AutoDoc_lib/Documentation_Title_Block
  motohawk_dout : MotoHawk_lib/Digital I//O Blocks/motohawk_dout  resource_name=[]  text_resource=FUELP  target=ECU555-80  use_diag=on  use_vardec=on  nam='FuelPumpPin'  read_level=1  write_level=1  group='System | I/O Pins | Outputs Config'
  motohawk_fault_def3 : MotoHawk_lib/Fault Management Blocks/motohawk_fault_def  nam='LSDFault_FuelPump'  mode=Enabled  id=[]  x=25  y=50  input_suspected=on  indeterminate=on  increment_xy=on  downsample_count=1  ovr_cals=on  ovr_actions=on  show_actions=off  action1_name=''  action1_cond=(None)  action2_name=''  action2_cond=(None)  action3_name=''  action3_cond=(None)  action4_name=''  action4_cond=(None)
  motohawk_probe6 : MotoHawk_lib/Calibration & Probing Blocks/motohawk_probe  nam='FuelPumpFaultState'  show_more=on  name_source=Use Parameter  read_level=1  view_as=Enumeration  enum_struct={'OK', 'Fault', 'Indeterminate'}  vector_style=Wide Row  use_help_units=on  help=''  units=''  use_header_enum=off  row_header_enum={}  col_header_enum={}  use_precision=off  precision=''  gain=1  offset=0  exp=1  use_mototune_group=on  mototune_group=motohawk_vardec_path(gcb)
  wires:
  State/1 -> Discrete Actuator - FUELP/1
  CompareTo/1 -> motohawk_fault_def3/1
  motohawk_dout/1 -> motohawk_probe6/1, CompareTo/1
  Discrete Actuator - FUELP/1 -> motohawk_dout/1
